simulink model "simulation_environment_thesis"  (R2024b)
settings: Solver=VariableStepAuto  StartTime=0.0  StopTime=3200  RelTol=1e-3
values from neighbouring files (symbols this model uses):
  A = [1, dt; 0, 1]    [estimate_and_control_fully_actuated_with_current.m]
  A1 = [1, 0, 0, 0, dt, 0 ... (first row only)    [estimate_and_control_fully_actuated_with_current.m]
  alpha = 10    [estimate_and_control_fully_actuated_with_current.m]
  alpha_xy_1 = 1    [estimate_and_control_fully_actuated_with_current.m]
  alpha_z_1 = 5    [estimate_and_control_fully_actuated_with_current.m]
  Az1 = [1, dt ... (first row only)    [estimate_and_control_fully_actuated_with_current.m]
  B = [dt; 0]    [estimate_and_control_fully_actuated_with_current.m]
  B1 = [dt, 0 ... (first row only)    [estimate_and_control_fully_actuated_with_current.m]
  Bz1 = [dt ... (first row only)    [estimate_and_control_fully_actuated_with_current.m]
  C1 = [1, 0, 0, 0, 0, 0 ... (first row only)    [estimate_and_control_fully_actuated_with_current.m]
  circumference_x = min_dist_from_ds*cos(theta)    [estimate_and_control_fully_actuated_with_current.m]
  circumference_y = min_dist_from_ds*sin(theta)    [estimate_and_control_fully_actuated_with_current.m]
  const_current = [-r; -s; 0; 0]    [estimate_and_control_fully_actuated_with_current.m]
  csi_psi_1 = 0.0003    [estimate_and_control_fully_actuated_with_current.m]
  csi_r_1 = 0.0003    [estimate_and_control_fully_actuated_with_current.m]
  csi_vcx_1 = 0.0003    [estimate_and_control_fully_actuated_with_current.m]
  csi_vcy_1 = 0.0003    [estimate_and_control_fully_actuated_with_current.m]
  csi_vcz_1 = 0.005    [estimate_and_control_fully_actuated_with_current.m]
  csi_x_1 = 0.003    [estimate_and_control_fully_actuated_with_current.m]
  csi_y_1 = 0.003    [estimate_and_control_fully_actuated_with_current.m]
  csi_z_1 = 0.005    [estimate_and_control_fully_actuated_with_current.m]
  Cz1 = [1, 0]    [estimate_and_control_fully_actuated_with_current.m]
  d = 1    [estimate_and_control_fully_actuated_with_current.m]
  delta_FOV = pi/7    (= 0.448798950513)    [estimate_and_control_fully_actuated_with_current.m]
  desired_speed = speed_0    [estimate_and_control_fully_actuated_with_current.m]
  dt = 1/Tsim    (= 0.005)    [estimate_and_control_fully_actuated_with_current.m]
  eta1 = deg2rad(0.5)    [estimate_and_control_fully_actuated_with_current.m]
  eta2 = deg2rad(0.5)    [estimate_and_control_fully_actuated_with_current.m]
  eta_psi_1 = 0.003    [estimate_and_control_fully_actuated_with_current.m]
  eta_x_1 = 0.03    [estimate_and_control_fully_actuated_with_current.m]
  eta_y_1 = 0.03    [estimate_and_control_fully_actuated_with_current.m]
  eta_z_1 = 0.005    [estimate_and_control_fully_actuated_with_current.m]
  G = dt*eye(2)    [estimate_and_control_fully_actuated_with_current.m]
  G1 = dt*eye(6)    [estimate_and_control_fully_actuated_with_current.m]
  Gz1 = dt*eye(2)    [estimate_and_control_fully_actuated_with_current.m]
  left_reference = L_x*[cos(NED_psi_ds); sin(NED_psi_ds); 0] + L_y_l*[-sin(NED_psi_ds); cos(NED_psi_ds); 0]    [estimate_and_control_fully_actuated_with_current.m]
  max_dist_from_ship = 60    [estimate_and_control_fully_actuated_with_current.m]
  min_dist_from_ds = 45    [estimate_and_control_fully_actuated_with_current.m]
  mu = -5    [estimate_and_control_fully_actuated_with_current.m]
  NED_psi_ds = -pi/2    (= -1.57079632679)    [estimate_and_control_fully_actuated_with_current.m]
  p_b1 = [-90; 90; 5]    [estimate_and_control_fully_actuated_with_current.m]
  position_t_0 = [0; 0; 0; 0]    [estimate_and_control_fully_actuated_with_current.m]
  psi_P = linspace(0,2*pi,360)'    [estimate_and_control_fully_actuated_with_current.m]
  Q = [csi1_kf^2, 0 ... (first row only)    [estimate_and_control_fully_actuated_with_current.m]
  Q1 = [csi_x_1_kf^2, 0, 0, 0, 0, 0 ... (first row only)    [estimate_and_control_fully_actuated_with_current.m]
  Qz1 = [csi_z_1_kf^2, 0 ... (first row only)    [estimate_and_control_fully_actuated_with_current.m]
  R = [eta1_kf^2, 0 ... (first row only)    [estimate_and_control_fully_actuated_with_current.m]
  R1 = [eta_x_1_kf^2, 0, 0 ... (first row only)    [estimate_and_control_fully_actuated_with_current.m]
  right_reference = L_x*[cos(NED_psi_ds); sin(NED_psi_ds); 0] + L_y_r*[sin(NED_psi_ds); -cos(NED_psi_ds); 0]    [estimate_and_control_fully_actuated_with_current.m]
  Rz1 = eta_z_1_kf^2    (= 1e-06)    [estimate_and_control_fully_actuated_with_current.m]
  speed_0 = [0.1, 0.1, 0, 0]'    [estimate_and_control_fully_actuated_with_current.m]
  tau_heave_sat = 30    [estimate_and_control_fully_actuated_with_current.m]
  tau_surge_sat = 10    [estimate_and_control_fully_actuated_with_current.m]
  tau_sway_sat = 30    [estimate_and_control_fully_actuated_with_current.m]
  tau_yaw_sat = 0.1    [estimate_and_control_fully_actuated_with_current.m]
  var_vm_x = 0.001    [estimate_and_control_fully_actuated_with_current.m]
  var_vm_y = 0.001    [estimate_and_control_fully_actuated_with_current.m]
  var_vm_z = 0.001    [estimate_and_control_fully_actuated_with_current.m]
undefined symbols (not defined in the file or in its folder): A2, alpha2, B2, csi1_2, csi2_2, csi3_2, csi4_2, csi5_2, csi6_2, csi7_2, csi8_2, eta1_2, eta2_2, eta3_2, eta4_2, eta5_2, eta6_2, G2, Q2, R2
libraries: simulink x60, simulink_extras x5
(part 14 of 16)

== matlab function simulation_environment_thesis/PHASE 3&4.22/PHASE  4/MATLAB Function4 ==
  data: yaw_curr input; enable output; yaw_dock input; i input; i output; yaw_dock output; enable input
function [enable, i, yaw_dock] = check_yaw_dock(yaw_curr, yaw_dock, i, enable)

    % enable = 0;
    % if abs(yaw_curr-yaw_prev)<= threshold
    %     enable = 1;
    % end


    N=100;
    N_start = 10000;
    % yaw_measured = zeros(N,1);
    if i>=N_start && i<N_start+N
        yaw_dock = yaw_curr;
        enable = 1;
    end
    % if i==N+N_start-1
    %     yaw_dock=mean(yaw_measured);
    %     enable = 1;
    % end
    i=i+1;

== matlab function simulation_environment_thesis/PHASE 3&4.22/PHASE  4/MATLAB Function5 ==
  data: angle input; angle output
function angle = normalize(angle)

angle = atan2(sin(angle), cos(angle));

== matlab function simulation_environment_thesis/PHASE 3&4.21/PHASE  3/dynamic + kinematic models1/MATLAB Function ==
  data: angle input; angle output
function angle = normalize(angle)

angle = atan2(sin(angle), cos(angle));

== matlab function simulation_environment_thesis/PHASE 3&4.21/PHASE  3/dynamic + kinematic models1/MATLAB Function7 ==
  data: speed_dot output; speed input; tau input
function speed_dot = dynamic_model(speed, tau) % speed_dot = speed_0_dot + delta_speed_dot = delta_speed_dot

    % speed and speed_dot are in the body frame
    speed_dot = zeros(4,1);
    
    W_plus_B = -0.8339;
    % m = 30;
    % I_z = 4;
    % X_du = -25;
    % Y_dv = -2;
    % Z_dw = - 20;
    % N_dr = -9;
    % X_u = -0.2;
    % Y_v = -55;
    % Z_w = 33; 
    % N_r = -4.14;
    % X_u_u = -20;
    % Y_v_v = -150;
    % Z_w_w = 90; 
    % N_r_r = -6.5;
    m = 30;
    I_z = 5;
    X_du = -25;
    Y_dv = -2;
    Z_dw = - 20;
    N_dr = -10;
    X_u = -0.2;
    Y_v = -55;
    Z_w = 35; 
    N_r = -5;
    X_u_u = -20;
    Y_v_v = -150;
    Z_w_w = 100; 
    N_r_r = -7;
    
    m_u = m - X_du;
    m_v = m - Y_dv;
    m_w = m - Z_dw;
    m_r = I_z - N_dr;
    m_u_v = m_u - m_v;
    
    % SIMPLIFIED MODEL
    
    % surge speed_dot
    speed_dot(1) = (X_u*speed(1) + X_u_u*speed(1)*abs(speed(1) + m_v*speed(2)*speed(4)) + tau(1))/m_u;
    % sway speed_dot
    speed_dot(2) = (Y_v*speed(2) + Y_v_v*speed(2)*abs(speed(2) - m_u*speed(1)*speed(4)) + tau(2))/m_v;
    % heave speed_dot
    speed_dot(3) = (-Z_w*speed(3) - Z_w_w*speed(3)*abs(speed(3)) + tau(3) + W_plus_B)/m_w;
    % yaw speed_dot
    speed_dot(4) = (N_r*speed(4) + N_r_r*speed(4)*abs(speed(4) + m_u_v*speed(1)*speed(2)) + tau(4))/m_r;

== matlab function simulation_environment_thesis/PHASE 3&4.21/PHASE  3/dynamic + kinematic models1/MATLAB Function9 ==
  data: yaw input; eta_dot output; speed input
function eta_dot = Q(yaw, speed) 
% yaw = orientation in the inertial frame, speed = robot's speed in body frame
% eta_dot = robot's speed in inertial frame

eta_dot = zeros(4,1);
R = [cos(yaw), -sin(yaw), 0;
    sin(yaw), cos(yaw), 0;
    0, 0, 1]; % z_inertial == z_body --> stesso frame, quindi la w corrisponde alla z_dot
eta_dot(1:3) = R*speed(1:3); 
eta_dot(4) = speed(4); % la yaw_dot == r di nuovo perché non ci sono né pitch né roll (stesso discorso della velocità lungo z)

== matlab function simulation_environment_thesis/PHASE 3&4.22/PHASE  4/CONTROLLER4/ANTIWINDUP SURGE/MATLAB Function21 ==
  data: in input; y output; K input
function y = fcn(in, K)
y = in*K;

== matlab function simulation_environment_thesis/PHASE 1.21/z, v_c_z estimate from DEPTH measurement/MATLAB Function8 ==
  data: K input; u input; y output
function y = fcn(K,u)

y = K*u;

== matlab function simulation_environment_thesis/PHASE 3&4.22/PHASE  4/CONTROLLER4/ANTIWINDUP SURGE/MATLAB Function22 ==
  data: in input; y output; K input
function y = fcn(in, K)
y = in*K;

== matlab function simulation_environment_thesis/PHASE 3&4.22/PHASE  4/CONTROLLER4/ANTIWINDUP SURGE/MATLAB Function23 ==
  data: e_s_u input; K_t_u input; y output
function y = AWU_surge(e_s_u, K_t_u)
y = K_t_u * e_s_u;

== matlab function simulation_environment_thesis/PHASE 3&4.22/PHASE  4/CONTROLLER4/ANTIWINDUP SWAY/MATLAB Function21 ==
  data: in input; y output; K input
function y = fcn(in, K)
y = in*K;

== matlab function simulation_environment_thesis/PHASE 3&4.22/PHASE  4/CONTROLLER4/ANTIWINDUP SWAY/MATLAB Function22 ==
  data: in input; y output; K input
function y = fcn(in, K)
y = in*K;

== matlab function simulation_environment_thesis/PHASE 3&4.22/PHASE  4/CONTROLLER4/ANTIWINDUP SWAY/MATLAB Function23 ==
  data: e_s_v input; K_t_v input; y output
function y = AWU_sway(e_s_v, K_t_v)
y = K_t_v * e_s_v;

== matlab function simulation_environment_thesis/PHASE 3&4.22/PHASE  4/CONTROLLER4/ANTIWINDUP_HEAVE/MATLAB Function11 ==
  data: e_s_w input; K_t_w input; y output
function y = AWU_heave(e_s_w, K_t_w)
y = K_t_w * e_s_w;

== matlab function simulation_environment_thesis/PHASE 3&4.22/PHASE  4/CONTROLLER4/ANTIWINDUP_HEAVE/MATLAB Function14 ==
  data: in input; y output; K input
function y = fcn(in, K)
y = in*K;

== matlab function simulation_environment_thesis/PHASE 3&4.22/PHASE  4/CONTROLLER4/ANTIWINDUP_HEAVE/MATLAB Function15 ==
  data: in input; y output; K input
function y = fcn(in, K)
y = in*K;

== matlab function simulation_environment_thesis/PHASE 3&4.22/PHASE  4/CONTROLLER4/ANTIWINDUP_HEAVE/MATLAB Function16 ==
  data: in input; y output; K input
function y = fcn(in, K)
y = in*K;

== matlab function simulation_environment_thesis/PHASE 3&4.22/PHASE  4/CONTROLLER4/ANTIWINDUP_YAW/MATLAB Function11 ==
  data: e_s_psi input; K_t_psi input; y output
function y = AWU_yaw(e_s_psi, K_t_psi)
y = K_t_psi * e_s_psi;

== matlab function simulation_environment_thesis/PHASE 1.21/z, v_c_z estimate from DEPTH measurement/MATLAB Function9 ==
  data: K_k output; P_k_k input; C input; P_k_k output; R input; A input; Q input; G input
function [K_k, P_k_k] = funct(P_k_k, C, R, A, Q, G)

P_knext_k = A*P_k_k*A' + G*Q*G';
K_k = P_knext_k*C'/(C*P_knext_k*C'+R);
P_k_k = (eye(2)-K_k*C)*P_knext_k;

== matlab function simulation_environment_thesis/PHASE 3&4.22/PHASE  4/CONTROLLER4/ANTIWINDUP_YAW/MATLAB Function14 ==
  data: in input; y output; K input
function y = fcn(in, K)
y = in*K;

== matlab function simulation_environment_thesis/PHASE 3&4.22/PHASE  4/CONTROLLER4/ANTIWINDUP_YAW/MATLAB Function15 ==
  data: in input; y output; K input
function y = fcn(in, K)
y = in*K;

== matlab function simulation_environment_thesis/PHASE 3&4.22/PHASE  4/CONTROLLER4/ANTIWINDUP_YAW/MATLAB Function16 ==
  data: in input; y output; K input
function y = fcn(in, K)
y = in*K;

== matlab function simulation_environment_thesis/PHASE 3&4.22/PHASE  4/CONTROLLER4/MATLAB Function3 ==
  data: K_i_u output; speed_0 input; K_p_u output; K_t_u output; K_i_v output; K_p_v output; K_t_v output; K_i_w output; K_p_w output; K_d_w output; K_t_w output; K_p_psi output; K_i_psi output; K_d_psi output; K_t_psi output
function [K_i_u, K_p_u, K_t_u, K_i_v, K_p_v, K_t_v, K_i_w, K_p_w, K_d_w, K_t_w, K_p_psi, K_i_psi, K_d_psi, K_t_psi] = computeGains(speed_0)
csi = 0.6;
omega_n = 0.2;
p = 10; % 10 o -10?

m = 30;
I_z = 4.14;
X_du = -25;
Y_dv = -2.325;
Z_dw = -19.4311;
N_dr = -8.690;
X_u = -0.2;
Y_v = -55.117;
Z_w = 33.6804; % CONTROLLA SEGNO con - viene positivo K_p, con + viene neg. dovrebbe essere positivo perché le eq sono al contrario (????)
X_u_u = -19.5;
Y_v_v = -147.9;
Z_w_w = 89.4105; % CONTROLLA SEGNO
N_r = -4.14;
N_r_r = -6.23;
m_u = m - X_du;
m_v = m - Y_dv;
m_w = m - Z_dw;
m_r = I_z - N_dr;

K_i_u = omega_n^2*m_u; %2
K_p_u = 2*csi*omega_n*m_u + X_u + X_u_u*2*abs(speed_0(1)); %9
t_i_u = K_p_u/K_i_u;
K_t_u = 1/t_i_u;


K_i_v = m_v*omega_n^2;
K_p_v = 2*csi*omega_n*m_v + Y_v + 2*Y_v_v*abs(speed_0(2));
t_i_v = K_p_v/K_i_v;
K_t_v = 1/t_i_v;

% PI SULLA VELOCITà SBAGLIATO
% K_i_w = m_w*omega_n^2; %2
% K_p_w = 2*csi*omega_n*m_w - Z_w - Z_w_w*2*abs(speed_0(3)); %negativo, -22
% t_i_w = K_p_w/K_i_w;
% K_t_w = 1/t_i_w;

% PID SULLA POSIZIONE
K_i_w = m_w*p*omega_n^2; % 19.7724
K_p_w = m_w*(omega_n^2 + 2*csi*omega_n*p); % 120.6119
K_d_w = m_w*(p + 2*csi*omega_n) - Z_w - Z_w_w*2*abs(speed_0(3)); % 472.4941
t_i_w = K_p_w/K_i_w;
t_d_w = K_d_w/K_p_w;
K_t_w = 1/sqrt(t_i_w*t_d_w);

K_p_psi = m_r*(omega_n^2 + 2*csi*omega_n*p); %31
K_i_psi = m_r*p*omega_n^2; %5
K_d_psi = m_r*(2*csi*omega_n + p) + N_r + N_r_r*2*abs(speed_0(4));
t_i_psi = K_p_psi/K_i_psi;
t_d_psi = K_d_psi/K_p_psi;
K_t_psi = 1/sqrt(t_i_psi*t_d_psi);

== matlab function simulation_environment_thesis/PHASE 3&4.22/PHASE  4/MATLAB Function ==
  data: ship_p_auv input; ds_p_auv output; ship_p_ds input
function ds_p_auv = coord_transf(ship_p_auv, ship_p_ds)


ds_p_auv = ship_p_auv - ship_p_ds;

== matlab function simulation_environment_thesis/PHASE 3&4.22/PHASE  4/MATLAB Function1 ==
  data: ship_p_auv output; ds_p_auv input; ship_p_ds input
function ship_p_auv = transform(ds_p_auv, ship_p_ds)
 

ship_p_auv = ship_p_ds + ds_p_auv;

== matlab function simulation_environment_thesis/PHASE 3&4.22/PHASE  4/MATLAB Function10 ==
  data: angle input; angle output
function angle = normalize(angle)

angle = atan2(sin(angle), cos(angle));

== matlab function simulation_environment_thesis/PHASE 3&4  /PHASE  4/dynamic + kinematic models1/MATLAB Function ==
  data: angle input; angle output
function angle = normalize(angle)

angle = atan2(sin(angle), cos(angle));

== matlab function simulation_environment_thesis/PHASE 3&4.22/PHASE  4/MATLAB Function18 ==
  data: yaw_err input; yaw_dock output; yaw_curr input
function yaw_dock = compute(yaw_err, yaw_curr)
    
    yaw_dock = yaw_curr - yaw_err;
    %yaw_dock = atan2(sin(yaw_dock), cos(yaw_dock));

== matlab function simulation_environment_thesis/PHASE 3&4  /PHASE  4/dynamic + kinematic models1/MATLAB Function7 ==
  data: speed_dot output; speed input; tau input
function speed_dot = dynamic_model(speed, tau) % speed_dot = speed_0_dot + delta_speed_dot = delta_speed_dot

    % speed and speed_dot are in the body frame
    speed_dot = zeros(4,1);
    
    W_plus_B = -0.8339;
    % m = 30;
    % I_z = 4;
    % X_du = -25;
    % Y_dv = -2;
    % Z_dw = - 20;
    % N_dr = -9;
    % X_u = -0.2;
    % Y_v = -55;
    % Z_w = 33; 
    % N_r = -4.14;
    % X_u_u = -20;
    % Y_v_v = -150;
    % Z_w_w = 90; 
    % N_r_r = -6.5;
    m = 30;
    I_z = 5;
    X_du = -25;
    Y_dv = -2;
    Z_dw = - 20;
    N_dr = -10;
    X_u = -0.2;
    Y_v = -55;
    Z_w = 35; 
    N_r = -5;
    X_u_u = -20;
    Y_v_v = -150;
    Z_w_w = 100; 
    N_r_r = -7;
    
    m_u = m - X_du;
    m_v = m - Y_dv;
    m_w = m - Z_dw;
    m_r = I_z - N_dr;
    m_u_v = m_u - m_v;
    
    % SIMPLIFIED MODEL
    
    % surge speed_dot
    speed_dot(1) = (X_u*speed(1) + X_u_u*speed(1)*abs(speed(1) + m_v*speed(2)*speed(4)) + tau(1))/m_u;
    % sway speed_dot
    speed_dot(2) = (Y_v*speed(2) + Y_v_v*speed(2)*abs(speed(2) - m_u*speed(1)*speed(4)) + tau(2))/m_v;
    % heave speed_dot
    speed_dot(3) = (-Z_w*speed(3) - Z_w_w*speed(3)*abs(speed(3)) + tau(3) + W_plus_B)/m_w;
    % yaw speed_dot
    speed_dot(4) = (N_r*speed(4) + N_r_r*speed(4)*abs(speed(4) + m_u_v*speed(1)*speed(2)) + tau(4))/m_r;

== matlab function simulation_environment_thesis/PHASE 3&4.22/PHASE  4/MATLAB Function6 ==
  data: angle input; angle output
function angle = normalize(angle)

angle = atan2(sin(angle), cos(angle));

== matlab function simulation_environment_thesis/PHASE 3&4.22/PHASE  4/MATLAB Function7 ==
  data: v_line input; v_blend output; v_circ input; w input
function v_blend = blend_vel(v_line, v_circ, w)

% K_perp = 0.5;
% t = [cos(NED_psi_dock); sin(NED_psi_dock)];
% n = [-t(2); t(1)];


v_blend = (1 - w) * v_circ + v_line;

== matlab function simulation_environment_thesis/PHASE 3&4.22/PHASE  4/MATLAB Function9 ==
  data: ds_p_auv input; docked output; ds_p_ds input; docked input; range output
function [docked, range] = check_distance(ds_p_auv, ds_p_ds, docked)
    
    threshold = 0.3; % 10 cm of threshold, but we can vary
    range = norm(ds_p_ds - ds_p_auv);
    if docked == 1
        return;
    end
    
    if range <= threshold
        docked = 1;
    end

== matlab function simulation_environment_thesis/PHASE 3&4  /PHASE  4/dynamic + kinematic models1/MATLAB Function9 ==
  data: yaw input; eta_dot output; speed input
function eta_dot = Q(yaw, speed) 
% yaw = orientation in the inertial frame, speed = robot's speed in body frame
% eta_dot = robot's speed in inertial frame

eta_dot = zeros(4,1);
R = [cos(yaw), -sin(yaw), 0;
    sin(yaw), cos(yaw), 0;
    0, 0, 1]; % z_inertial == z_body --> stesso frame, quindi la w corrisponde alla z_dot
eta_dot(1:3) = R*speed(1:3); 
eta_dot(4) = speed(4); % la yaw_dot == r di nuovo perché non ci sono né pitch né roll (stesso discorso della velocità lungo z)

== matlab function simulation_environment_thesis/PHASE 3&4.22/PHASE  4/Subsystem/MATLAB Function1 ==
  data: radius input; stop_flag output; NED_psi_dock input; v_c input; t_trans input; x_y input; stop_flag input; ds_Pstart output; ds_Pstart_prev output; dist output
function [stop_flag, ds_Pstart, ds_Pstart_prev, dist] = check_distance(radius, NED_psi_dock, v_c, t_trans, x_y, stop_flag)
    
    threshold = 5;
    NED_psi_ds = NED_psi_dock - pi;
    ds_Pstart_prev = radius*[cos(NED_psi_ds); sin(NED_psi_ds)];
    ds_drift = v_c*t_trans;
    ds_Pstart = ds_Pstart_prev - ds_drift;
    dist = ds_Pstart - x_y;
    dist = abs(dist);
    if stop_flag == 1
        return;
    end
    
    if dist <= threshold*ones(2,1)
        stop_flag = 1;
    end

== matlab function simulation_environment_thesis/PHASE 1.22/CONTROLLER1/ANTIWINDUP SURGE/MATLAB Function21 ==
  data: in input; y output; K input
function y = fcn(in, K)
y = in*K;

== matlab function simulation_environment_thesis/PHASE 3&4.22/PHASE  4/Subsystem/MATLAB Function2 ==
  data: right_trans input; stop_flag output; left_trans input; ds_p_auv input; stop_flag input; P_R_range output; P_L_range output
function [stop_flag, P_R_range, P_L_range] = check_distance(right_trans, left_trans, ds_p_auv, stop_flag)
    
    
    threshold = 0.01; % vediamo se basta
    P_R_range = norm(right_trans(1:2) - ds_p_auv);
    P_L_range = norm(left_trans(1:2) - ds_p_auv);
    if stop_flag == 1
        return;
    end
    if abs(P_R_range - P_L_range) <= threshold
        stop_flag = 1;
    end

== matlab function simulation_environment_thesis/PHASE 3&4.22/PHASE  4/Subsystem/MATLAB Function3 ==
  data: v_tot_des input; v_c input; psi_dock input; speed0 input; speed_desired output; beta input
function speed_desired = circ_guidance(v_tot_des, v_c, psi_dock, speed0, beta)
    v_c_x = v_c(1);
    v_c_y = v_c(2);
    
    
    
    u = cos(psi_dock)*(v_tot_des*cos(beta) - v_c_x) + sin(psi_dock)*(v_tot_des*sin(beta) - v_c_y);
    v = -sin(psi_dock)*(v_tot_des*cos(beta) - v_c_x) + cos(psi_dock)*(v_tot_des*sin(beta) - v_c_y);
    speed_desired = [u; v; speed0(3:4)];

== matlab function simulation_environment_thesis/PHASE 3&4.22/PHASE  4/Subsystem/MATLAB Function4 ==
  data: NED_psi_dock input; t output
function t = tang(NED_psi_dock)

    t = [cos(NED_psi_dock); sin(NED_psi_dock)];

== matlab function simulation_environment_thesis/PHASE 1.22/dynamic + kinematic models1/MATLAB Function ==
  data: angle input; angle output
function angle = normalize(angle)

angle = atan2(sin(angle), cos(angle));

== matlab function simulation_environment_thesis/PHASE 1.22/dynamic + kinematic models1/MATLAB Function7 ==
  data: speed_dot output; speed input; tau input
function speed_dot = dynamic_model(speed, tau) % speed_dot = speed_0_dot + delta_speed_dot = delta_speed_dot

    % speed and speed_dot are in the body frame
    speed_dot = zeros(4,1);
    
    W_plus_B = -0.8339;
    % m = 30;
    % I_z = 4;
    % X_du = -25;
    % Y_dv = -2;
    % Z_dw = - 20;
    % N_dr = -9;
    % X_u = -0.2;
    % Y_v = -55;
    % Z_w = 33; 
    % N_r = -4.14;
    % X_u_u = -20;
    % Y_v_v = -150;
    % Z_w_w = 90; 
    % N_r_r = -6.5;
    m = 30;
    I_z = 5;
    X_du = -25;
    Y_dv = -2;
    Z_dw = - 20;
    N_dr = -10;
    X_u = -0.2;
    Y_v = -55;
    Z_w = 35; 
    N_r = -5;
    X_u_u = -20;
    Y_v_v = -150;
    Z_w_w = 100; 
    N_r_r = -7;
    
    m_u = m - X_du;
    m_v = m - Y_dv;
    m_w = m - Z_dw;
    m_r = I_z - N_dr;
    m_u_v = m_u - m_v;
    
    % SIMPLIFIED MODEL
    
    % surge speed_dot
    speed_dot(1) = (X_u*speed(1) + X_u_u*speed(1)*abs(speed(1) + m_v*speed(2)*speed(4)) + tau(1))/m_u;
    % sway speed_dot
    speed_dot(2) = (Y_v*speed(2) + Y_v_v*speed(2)*abs(speed(2) - m_u*speed(1)*speed(4)) + tau(2))/m_v;
    % heave speed_dot
    speed_dot(3) = (-Z_w*speed(3) - Z_w_w*speed(3)*abs(speed(3)) + tau(3) + W_plus_B)/m_w;
    % yaw speed_dot
    speed_dot(4) = (N_r*speed(4) + N_r_r*speed(4)*abs(speed(4) + m_u_v*speed(1)*speed(2)) + tau(4))/m_r;

== matlab function simulation_environment_thesis/PHASE 3&4.22/PHASE  4/Subsystem/MATLAB Function7 ==
  data: eta input; circ_x input; circ_y input; psi_P input; beta input; direction output; flag output; psi_P output; i output
function [direction, flag, psi_P, i]= closest_pnt(eta, circ_x, circ_y, psi_P, beta)
    n = size(circ_x,1);
    p_r = eta(1:2, 1); % OK
    eucl_dist = zeros(n,1);
    for j = 1:n
        eucl_dist(j) = sqrt((p_r(1)-circ_x(j))^2+(p_r(2)-circ_y(j))^2);
    end
    [min_dist, i] = min(eucl_dist);
    flag = 0; % to see if there's a 2pi gap between the previous psi and the current one

    if abs(psi_P(i)-beta)> 2*pi
        flag = 1;
        psi_P = psi_P - 2*pi; % controlla la situa con - o col +, potrebbe esserci un problema se non devo sottrarre ma sommare 2pi
    end
    direction = psi_P(i) - pi/2;
    direction = atan2(sin(direction), cos(direction));
    end

== matlab function simulation_environment_thesis/PHASE 3&4.22/PHASE  4/MATLAB Function8 ==
  data: u input; y output
function y = fcn(u)

y = u;
if abs(u-2*pi)<=0.01
    y = u-2*pi;
elseif abs(u+2*pi)<=0.01
    y = u+2*pi;
end

== matlab function simulation_environment_thesis/PHASE 1.24/dynamic + kinematic models1/MATLAB Function ==
  data: angle input; angle output
function angle = normalize(angle)

angle = atan2(sin(angle), cos(angle));

== matlab function simulation_environment_thesis/PHASE 1.22/CONTROLLER1/ANTIWINDUP SURGE/MATLAB Function22 ==
  data: in input; y output; K input
function y = fcn(in, K)
y = in*K;

== matlab function simulation_environment_thesis/PHASE 1.24/dynamic + kinematic models1/MATLAB Function7 ==
  data: speed_dot output; speed input; tau input
function speed_dot = dynamic_model(speed, tau) % speed_dot = speed_0_dot + delta_speed_dot = delta_speed_dot

    % speed and speed_dot are in the body frame
    speed_dot = zeros(4,1);
    
    W_plus_B = -0.8339;
    % m = 30;
    % I_z = 4;
    % X_du = -25;
    % Y_dv = -2;
    % Z_dw = - 20;
    % N_dr = -9;
    % X_u = -0.2;
    % Y_v = -55;
    % Z_w = 33; 
    % N_r = -4.14;
    % X_u_u = -20;
    % Y_v_v = -150;
    % Z_w_w = 90; 
    % N_r_r = -6.5;
    m = 30;
    I_z = 5;
    X_du = -25;
    Y_dv = -2;
    Z_dw = - 20;
    N_dr = -10;
    X_u = -0.2;
    Y_v = -55;
    Z_w = 35; 
    N_r = -5;
    X_u_u = -20;
    Y_v_v = -150;
    Z_w_w = 100; 
    N_r_r = -7;
    
    m_u = m - X_du;
    m_v = m - Y_dv;
    m_w = m - Z_dw;
    m_r = I_z - N_dr;
    m_u_v = m_u - m_v;
    
    % SIMPLIFIED MODEL
    
    % surge speed_dot
    speed_dot(1) = (X_u*speed(1) + X_u_u*speed(1)*abs(speed(1) + m_v*speed(2)*speed(4)) + tau(1))/m_u;
    % sway speed_dot
    speed_dot(2) = (Y_v*speed(2) + Y_v_v*speed(2)*abs(speed(2) - m_u*speed(1)*speed(4)) + tau(2))/m_v;
    % heave speed_dot
    speed_dot(3) = (-Z_w*speed(3) - Z_w_w*speed(3)*abs(speed(3)) + tau(3) + W_plus_B)/m_w;
    % yaw speed_dot
    speed_dot(4) = (N_r*speed(4) + N_r_r*speed(4)*abs(speed(4) + m_u_v*speed(1)*speed(2)) + tau(4))/m_r;

== matlab function simulation_environment_thesis/PHASE 3&4.22/PHASE  4/p, v_c, psi, r (3D) estimate, from RANGE, v_c, PSI measurement/MATLAB Function ==
  data: p input; y output
function y = range(p)
    y = sqrt(p(1)^2 + p(2)^2 + p(3)^2);

== matlab function simulation_environment_thesis/PHASE 3&4.22/PHASE  4/p, v_c, psi, r (3D) estimate, from RANGE, v_c, PSI measurement/MATLAB Function10 ==
  data: K_knext output; P_k_k input; P_knext_knext output; R input; A input; Q input; G input; x_k_kprev input
function [K_knext, P_knext_knext] = funct(P_k_k, R, A, Q, G, x_k_kprev)

P_knext_k = A*P_k_k*A' + G*Q*G';
H = zeros(6,8);
H(1,1) = x_k_kprev(1)/sqrt(x_k_kprev(1)^2+x_k_kprev(2)^2+x_k_kprev(3)^2);
H(1,2) = x_k_kprev(2)/sqrt(x_k_kprev(1)^2+x_k_kprev(2)^2+x_k_kprev(3)^2);
H(1,3) = x_k_kprev(3)/sqrt(x_k_kprev(1)^2+x_k_kprev(2)^2+x_k_kprev(3)^2);
H(2,4) = 1;
H(3,5) = 1;
H(4,6) = 1;
H(5,7) = 1;
H(6,3) = 1;
K_knext = P_knext_k*H'/(H*P_knext_k*H'+R);
P_knext_knext = (eye(8)-K_knext*H)*P_knext_k;

== matlab function simulation_environment_thesis/PHASE 3&4.22/PHASE  4/p, v_c, psi, r (3D) estimate, from RANGE, v_c, PSI measurement/MATLAB Function6 ==
  data: x_k_kprev input; y output
function y = pred(x_k_kprev)

y = zeros(6,1);
y(1) =sqrt(x_k_kprev(1)^2 + x_k_kprev(2)^2 + x_k_kprev(3)^2);
y(2:5) = x_k_kprev(4:7);
y(6) = x_k_kprev(3);

== matlab function simulation_environment_thesis/PHASE 3&4.22/PHASE  4/p, v_c, psi, r (3D) estimate, from RANGE, v_c, PSI measurement/MATLAB Function7 ==
  data: A input; u input; x_k_k output
function x_k_k = fcn(A,u)

x_k_k = A*u;

== matlab function simulation_environment_thesis/PHASE 3&4.22/PHASE  4/p, v_c, psi, r (3D) estimate, from RANGE, v_c, PSI measurement/MATLAB Function8 ==
  data: B input; u input; y output
function y = fcn(B,u)

y = B*u;

== matlab function simulation_environment_thesis/PHASE 3&4.22/PHASE  4/p, v_c, psi, r (3D) estimate, from RANGE, v_c, PSI measurement/MATLAB Function9 ==
  data: K input; y_err input; y output
function y = err(K,y_err)

y = K*y_err;

== matlab function simulation_environment_thesis/PHASE 3&4.22/PHASE  4/positon and current estimate, XY PLANE from X, Y, psi measurement/MATLAB Function10 ==
  data: K_knext output; P_k_k input; P_knext_knext output; R input; A input; Q input; G input; C input
function [K_knext, P_knext_knext] = funct(P_k_k, R, A, Q, G, C)

P_knext_k = A*P_k_k*A' + G*Q*G';
K_knext = P_knext_k*C'/(C*P_knext_k*C'+R);
P_knext_knext = (eye(6)-K_knext*C)*P_knext_k;

== matlab function simulation_environment_thesis/PHASE 3&4.22/PHASE  4/positon and current estimate, XY PLANE from X, Y, psi measurement/MATLAB Function6 ==
  data: x_k_kprev input; pred output; C input
function pred = fcn(x_k_kprev, C)
pred = C*x_k_kprev;

== matlab function simulation_environment_thesis/PHASE 1.22/CONTROLLER1/ANTIWINDUP SURGE/MATLAB Function23 ==
  data: e_s_u input; K_t_u input; y output
function y = AWU_surge(e_s_u, K_t_u)
y = K_t_u * e_s_u;

== matlab function simulation_environment_thesis/PHASE 3&4.22/PHASE  4/positon and current estimate, XY PLANE from X, Y, psi measurement/MATLAB Function7 ==
  data: A input; u input; x_k_k output
function x_k_k = fcn(A,u)

x_k_k = A*u;

== matlab function simulation_environment_thesis/PHASE 3&4.22/PHASE  4/positon and current estimate, XY PLANE from X, Y, psi measurement/MATLAB Function8 ==
  data: B input; u input; y output
function y = fcn(B,u)

y = B*u;

== matlab function simulation_environment_thesis/PHASE 3&4.22/PHASE  4/positon and current estimate, XY PLANE from X, Y, psi measurement/MATLAB Function9 ==
  data: K input; y_err input; y output
function y = fcn(K,y_err)

y = K*y_err;

== matlab function simulation_environment_thesis/PHASE 3&4.22/PHASE  4/relative yaw//bias estimate from VECTOR measurement1/MATLAB Function12 ==
  data: d input; psi input; y output
function y = output_est(d, psi)
 
y = zeros(2,1);
y(1) = - d*sin(psi);
y(2) = d*cos(psi);

== matlab function simulation_environment_thesis/PHASE 3&4.22/PHASE  4/relative yaw//bias estimate from VECTOR measurement1/MATLAB Function13 ==
  data: K_k output; P_k_k input; R input; A input; Q input; G input; x_k_kprev input; P_k_k output; d input
function [K_k, P_k_k] = funct(P_k_k, R, A, Q, G, x_k_kprev, d)

P_knext_k = A*P_k_k*A' + G*Q*G';
H = zeros(2);
H(1,1) = - d*cos(x_k_kprev(1));
H(2,1) = - d*sin(x_k_kprev(1));

K_k = P_knext_k*H'/(H*P_knext_k*H'+R);
P_k_k = (eye(2)-K_k*H)*P_knext_k;

== matlab function simulation_environment_thesis/PHASE 3&4.22/PHASE  4/relative yaw//bias estimate from VECTOR measurement1/MATLAB Function14 ==
  data: A input; u input; y output
function y = fcn(A,u)

y = A*u;

== matlab function simulation_environment_thesis/PHASE 3&4.22/PHASE  4/relative yaw//bias estimate from VECTOR measurement1/MATLAB Function15 ==
  data: K input; u input; y output
function y = err(K,u)

y = K*u;

== matlab function simulation_environment_thesis/PHASE 3&4.22/PHASE  4/relative yaw//bias estimate from VECTOR measurement1/MATLAB Function16 ==
  data: B input; u input; y output
function y = fcn(B,u)

y = B*u;

== matlab function simulation_environment_thesis/PHASE 3&4.22/PHASE  4/relative yaw//bias estimate from VECTOR measurement1/MATLAB Function3 ==
  data: psi input; y output; d input
function y = real_obs(psi, d)

y = zeros(2,1);
y(1) = - d*sin(psi);
y(2) = d*cos(psi);

== matlab function simulation_environment_thesis/PHASE 1.22/CONTROLLER1/ANTIWINDUP SWAY/MATLAB Function21 ==
  data: in input; y output; K input
function y = fcn(in, K)
y = in*K;

== matlab function simulation_environment_thesis/PHASE 3&4.22/Subsystem3/MATLAB Function7 ==
  data: num input; out output
function out = phase(num)

    out = NaN;
    if num == 1
        out = 0;
    elseif num == 2
        out = 1;
    end

== matlab function simulation_environment_thesis/PHASE 3&4.22/end phase 3/# of pings ==
  data: alpha_ds input; num_of_pings output; delta input; ds_p_ds input; ds_p_auv input
function num_of_pings = sensor(alpha_ds, delta, ds_p_ds, ds_p_auv)

    dir = [cos(alpha_ds), sin(alpha_ds)]; % main direction of the DS opening cone
    distance = ds_p_auv(1:2) - ds_p_ds; % vector connecting the center of the DS to the AUV
    dot_prod = dot(distance,dir) / norm(distance);

    in_cone = (dot_prod >= cos(delta)) & (norm(distance) > 0);

    if in_cone == 0 % go to PHASE 3
        num_of_pings = 1; %% come gestisco quando ne tornano 0? ne possono tornare 0?
    else % go to PHASE 4
        num_of_pings = 2;
    end

== matlab function simulation_environment_thesis/PHASE 3&4.22/logic to recognize where i am, based on the # of pings/MATLAB Function6 ==
  data: num input; out output
function out = phase(num)
    out = 0;
    if num == 1
        out = 0;
    elseif num == 2
        out = 1;
    end

== matlab function simulation_environment_thesis/PHASE 3&4.22/simulator of pings from sensor/# of pings ==
  data: alpha_ds input; num_of_pings output; delta input; ds_p_ds input; ds_p_auv input; stop_flag input
function num_of_pings = sensor(alpha_ds, delta, ds_p_ds, ds_p_auv, stop_flag)
    if stop_flag == 0 
        num_of_pings = NaN;
        return;
    end

    dir = [cos(alpha_ds), sin(alpha_ds)]; % main direction of the DS opening cone
    distance = ds_p_auv(1:2) - ds_p_ds; % vector connecting the center of the DS to the AUV
    dot_prod = dot(distance,dir) / norm(distance);

    in_cone = (dot_prod >= cos(delta)) & (norm(distance) > 0);

    if in_cone == 0 % go to PHASE 3
        num_of_pings = 1; %% come gestisco quando ne tornano 0? ne possono tornare 0?
    else % go to PHASE 4
        num_of_pings = 2;
    end

== matlab function simulation_environment_thesis/PHASE 3&4  /PHASE  3/CONTROLLER/ANTIWINDUP SURGE/MATLAB Function21 ==
  data: in input; y output; K input
function y = fcn(in, K)
y = in*K;

== matlab function simulation_environment_thesis/PHASE 3&4  /PHASE  3/CONTROLLER/ANTIWINDUP SURGE/MATLAB Function22 ==
  data: in input; y output; K input
function y = fcn(in, K)
y = in*K;

== matlab function simulation_environment_thesis/PHASE 3&4  /PHASE  3/CONTROLLER/ANTIWINDUP SURGE/MATLAB Function23 ==
  data: e_s_u input; K_t_u input; y output
function y = AWU_surge(e_s_u, K_t_u)
y = K_t_u * e_s_u;

== matlab function simulation_environment_thesis/PHASE 3&4  /PHASE  3/CONTROLLER/ANTIWINDUP SWAY/MATLAB Function21 ==
  data: in input; y output; K input
function y = fcn(in, K)
y = in*K;

== matlab function simulation_environment_thesis/PHASE 3&4  /PHASE  3/CONTROLLER/ANTIWINDUP SWAY/MATLAB Function22 ==
  data: in input; y output; K input
function y = fcn(in, K)
y = in*K;

== matlab function simulation_environment_thesis/PHASE 3&4  /PHASE  3/CONTROLLER/ANTIWINDUP SWAY/MATLAB Function23 ==
  data: e_s_v input; K_t_v input; y output
function y = AWU_sway(e_s_v, K_t_v)
y = K_t_v * e_s_v;

== matlab function simulation_environment_thesis/PHASE 1.22/CONTROLLER1/ANTIWINDUP SWAY/MATLAB Function22 ==
  data: in input; y output; K input
function y = fcn(in, K)
y = in*K;

== matlab function simulation_environment_thesis/PHASE 3&4  /PHASE  3/CONTROLLER/ANTIWINDUP_HEAVE/MATLAB Function11 ==
  data: e_s_w input; K_t_w input; y output
function y = AWU_heave(e_s_w, K_t_w)
y = K_t_w * e_s_w;

== matlab function simulation_environment_thesis/PHASE 3&4  /PHASE  3/CONTROLLER/ANTIWINDUP_HEAVE/MATLAB Function14 ==
  data: in input; y output; K input
function y = fcn(in, K)
y = in*K;

== matlab function simulation_environment_thesis/PHASE 3&4  /PHASE  3/CONTROLLER/ANTIWINDUP_HEAVE/MATLAB Function15 ==
  data: in input; y output; K input
function y = fcn(in, K)
y = in*K;

== matlab function simulation_environment_thesis/PHASE 3&4  /PHASE  3/CONTROLLER/ANTIWINDUP_HEAVE/MATLAB Function16 ==
  data: in input; y output; K input
function y = fcn(in, K)
y = in*K;

== matlab function simulation_environment_thesis/PHASE 3&4  /PHASE  3/CONTROLLER/ANTIWINDUP_YAW/MATLAB Function11 ==
  data: e_s_psi input; K_t_psi input; y output
function y = AWU_yaw(e_s_psi, K_t_psi)
y = K_t_psi * e_s_psi;

== matlab function simulation_environment_thesis/PHASE 3&4  /PHASE  3/CONTROLLER/ANTIWINDUP_YAW/MATLAB Function14 ==
  data: in input; y output; K input
function y = fcn(in, K)
y = in*K;

== matlab function simulation_environment_thesis/PHASE 3&4  /PHASE  3/CONTROLLER/ANTIWINDUP_YAW/MATLAB Function15 ==
  data: in input; y output; K input
function y = fcn(in, K)
y = in*K;

== matlab function simulation_environment_thesis/PHASE 3&4  /PHASE  3/CONTROLLER/ANTIWINDUP_YAW/MATLAB Function16 ==
  data: in input; y output; K input
function y = fcn(in, K)
y = in*K;

== matlab function simulation_environment_thesis/PHASE 3&4  /PHASE  3/CONTROLLER/MATLAB Function3 ==
  data: K_i_u output; speed_0 input; K_p_u output; K_t_u output; K_i_v output; K_p_v output; K_t_v output; K_i_w output; K_p_w output; K_d_w output; K_t_w output; K_p_psi output; K_i_psi output; K_d_psi output; K_t_psi output
function [K_i_u, K_p_u, K_t_u, K_i_v, K_p_v, K_t_v, K_i_w, K_p_w, K_d_w, K_t_w, K_p_psi, K_i_psi, K_d_psi, K_t_psi] = computeGains(speed_0)
csi = 0.6;
omega_n = 0.2;
p = 10; % 10 o -10?

m = 30;
I_z = 4.14;
X_du = -25;
Y_dv = -2.325;
Z_dw = -19.4311;
N_dr = -8.690;
X_u = -0.2;
Y_v = -55.117;
Z_w = 33.6804; % CONTROLLA SEGNO con - viene positivo K_p, con + viene neg. dovrebbe essere positivo perché le eq sono al contrario (????)
X_u_u = -19.5;
Y_v_v = -147.9;
Z_w_w = 89.4105; % CONTROLLA SEGNO
N_r = -4.14;
N_r_r = -6.23;
m_u = m - X_du;
m_v = m - Y_dv;
m_w = m - Z_dw;
m_r = I_z - N_dr;

K_i_u = omega_n^2*m_u; %2
K_p_u = 2*csi*omega_n*m_u + X_u + X_u_u*2*abs(speed_0(1)); %9
t_i_u = K_p_u/K_i_u;
K_t_u = 1/t_i_u;


K_i_v = m_v*omega_n^2;
K_p_v = 2*csi*omega_n*m_v + Y_v + 2*Y_v_v*abs(speed_0(2));
t_i_v = K_p_v/K_i_v;
K_t_v = 1/t_i_v;

% PI SULLA VELOCITà SBAGLIATO
% K_i_w = m_w*omega_n^2; %2
% K_p_w = 2*csi*omega_n*m_w - Z_w - Z_w_w*2*abs(speed_0(3)); %negativo, -22
% t_i_w = K_p_w/K_i_w;
% K_t_w = 1/t_i_w;

% PID SULLA POSIZIONE
K_i_w = m_w*p*omega_n^2; % 19.7724
K_p_w = m_w*(omega_n^2 + 2*csi*omega_n*p); % 120.6119
K_d_w = m_w*(p + 2*csi*omega_n) - Z_w - Z_w_w*2*abs(speed_0(3)); % 472.4941
t_i_w = K_p_w/K_i_w;
t_d_w = K_d_w/K_p_w;
K_t_w = 1/sqrt(t_i_w*t_d_w);

K_p_psi = m_r*(omega_n^2 + 2*csi*omega_n*p); %31
K_i_psi = m_r*p*omega_n^2; %5
K_d_psi = m_r*(2*csi*omega_n + p) + N_r + N_r_r*2*abs(speed_0(4));
t_i_psi = K_p_psi/K_i_psi;
t_d_psi = K_d_psi/K_p_psi;
K_t_psi = 1/sqrt(t_i_psi*t_d_psi);

== matlab function simulation_environment_thesis/PHASE 1.22/CONTROLLER1/ANTIWINDUP SWAY/MATLAB Function23 ==
  data: e_s_v input; K_t_v input; y output
function y = AWU_sway(e_s_v, K_t_v)
y = K_t_v * e_s_v;

== matlab function simulation_environment_thesis/PHASE 3&4  /PHASE  3/MATLAB Function ==
  data: ship_p_auv input; ds_p_auv output; ship_p_ds input
function ds_p_auv = coord_transf(ship_p_auv, ship_p_ds)


ds_p_auv = ship_p_auv - ship_p_ds;

== matlab function simulation_environment_thesis/PHASE 3&4  /PHASE  3/MATLAB Function1 ==
  data: ship_p_auv output; ds_p_auv input; ship_p_ds input
function ship_p_auv = fcn(ds_p_auv, ship_p_ds)
 

ship_p_auv = ship_p_ds + ds_p_auv;

== matlab function simulation_environment_thesis/PHASE 1.22/dynamic + kinematic models1/MATLAB Function9 ==
  data: yaw input; eta_dot output; speed input
function eta_dot = Q(yaw, speed) 
% yaw = orientation in the inertial frame, speed = robot's speed in body frame
% eta_dot = robot's speed in inertial frame

eta_dot = zeros(4,1);
R = [cos(yaw), -sin(yaw), 0;
    sin(yaw), cos(yaw), 0;
    0, 0, 1]; % z_inertial == z_body --> stesso frame, quindi la w corrisponde alla z_dot
eta_dot(1:3) = R*speed(1:3); 
eta_dot(4) = speed(4); % la yaw_dot == r di nuovo perché non ci sono né pitch né roll (stesso discorso della velocità lungo z)

== matlab function simulation_environment_thesis/PHASE 3&4  /PHASE  3/REFERENCE GENERATOR/MATLAB Function2 ==
  data: yaw_des input; yaw_des output
function yaw_des = normalize(yaw_des)

yaw_des = atan2(sin(yaw_des), cos(yaw_des));

== matlab function simulation_environment_thesis/PHASE 3&4  /PHASE  3/REFERENCE GENERATOR/MATLAB Function3 ==
  data: psi_p input; psi output; y_1 input; delta_h input
function psi = heading_ctrl_law(psi_p, y_1, delta_h)
    psi = psi_p + atan2(y_1,delta_h);
    % no -y_1 because of how I built y_1

== matlab function simulation_environment_thesis/PHASE 3&4  /PHASE  3/REFERENCE GENERATOR/MATLAB Function4 ==
  data: y_1 output; eta input; circ_x input; circ_y input; center input; radius input; psi_P input; prev_psi_P input; direction output; flag output; psi_P output; i output
function [y_1, direction, flag, psi_P, i]= closest_pnt(eta, circ_x, circ_y, center, radius, psi_P, prev_psi_P)
    n = size(circ_x,1);
    p_r = eta(1:2, 1); % OK
    eucl_dist = zeros(n,1);
    for j = 1:n
        eucl_dist(j) = sqrt((p_r(1)-circ_x(j))^2+(p_r(2)-circ_y(j))^2);
    end
    [min_dist, i] = min(eucl_dist);


    closest = [circ_x(i); circ_y(i)]; % OK
    y_1_I = p_r - closest; % OK
    y_1 = sqrt(y_1_I(1)^2 + y_1_I(2)^2); % OK
    sign = 1;
    dist = p_r - center;
    dist_mod = sqrt(dist(1)^2 + dist(2)^2);
    if dist_mod > radius
        sign = -1;
    elseif abs(dist_mod - radius) < 0.01
        sign = 0;
    end
    flag = 0; % to see if there's a 2pi gap between the previous psi and the current one
    y_1 = y_1*sign;

    if abs(psi_P(i)-prev_psi_P)> 2*pi
        flag = 1;
        psi_P = psi_P - 2*pi; % controlla la situa con - o col +, potrebbe esserci un problema se non devo sottrarre ma sommare 2pi
    end
    direction = psi_P(i) - pi/2;
    direction = atan2(sin(direction), cos(direction));
    end

== matlab function simulation_environment_thesis/PHASE 3&4  /PHASE  3/REFERENCE GENERATOR/MATLAB Function6 ==
  data: yaw_ref input; yaw_error output; yaw_est input
function yaw_error = normalize(yaw_ref, yaw_est)

yaw_error = atan2(sin(yaw_ref - yaw_est), cos(yaw_ref - yaw_est));

== matlab function simulation_environment_thesis/PHASE 3&4  /PHASE  3/REFERENCE GENERATOR/MATLAB Function7 ==
  data: v_c input; speed output; beta input; speed_0 input; yaw_dock input
function speed = get_yaw(v_c, beta, speed_0, yaw_dock)

    speed = zeros(4,1);
    speed(3:4) = speed_0(3:4);
    v_tot = norm(speed_0);

    speed(1) = cos(yaw_dock)*(v_tot*cos(beta) - v_c(1)) + sin(yaw_dock)*(v_tot*sin(beta) - v_c(2));
    speed(2) = -sin(yaw_dock)*(v_tot*cos(beta) - v_c(1)) + cos(yaw_dock)*(v_tot*sin(beta) - v_c(2));

    
end

== matlab function simulation_environment_thesis/PHASE 1.22/CONTROLLER1/ANTIWINDUP_HEAVE/MATLAB Function11 ==
  data: e_s_w input; K_t_w input; y output
function y = AWU_heave(e_s_w, K_t_w)
y = K_t_w * e_s_w;

== matlab function simulation_environment_thesis/PHASE 1.24/dynamic + kinematic models1/MATLAB Function9 ==
  data: yaw input; eta_dot output; speed input
function eta_dot = Q(yaw, speed) 
% yaw = orientation in the inertial frame, speed = robot's speed in body frame
% eta_dot = robot's speed in inertial frame

eta_dot = zeros(4,1);
R = [cos(yaw), -sin(yaw), 0;
    sin(yaw), cos(yaw), 0;
    0, 0, 1]; % z_inertial == z_body --> stesso frame, quindi la w corrisponde alla z_dot
eta_dot(1:3) = R*speed(1:3); 
eta_dot(4) = speed(4); % la yaw_dot == r di nuovo perché non ci sono né pitch né roll (stesso discorso della velocità lungo z)

== matlab function simulation_environment_thesis/PHASE 3&4.24/PHASE  4/dynamic + kinematic models1/MATLAB Function ==
  data: angle input; angle output
function angle = normalize(angle)

angle = atan2(sin(angle), cos(angle));

== matlab function simulation_environment_thesis/PHASE 3&4.24/PHASE  4/dynamic + kinematic models1/MATLAB Function7 ==
  data: speed_dot output; speed input; tau input
function speed_dot = dynamic_model(speed, tau) % speed_dot = speed_0_dot + delta_speed_dot = delta_speed_dot

    % speed and speed_dot are in the body frame
    speed_dot = zeros(4,1);
    
    W_plus_B = -0.8339;
    % m = 30;
    % I_z = 4;
    % X_du = -25;
    % Y_dv = -2;
    % Z_dw = - 20;
    % N_dr = -9;
    % X_u = -0.2;
    % Y_v = -55;
    % Z_w = 33; 
    % N_r = -4.14;
    % X_u_u = -20;
    % Y_v_v = -150;
    % Z_w_w = 90; 
    % N_r_r = -6.5;
    m = 30;
    I_z = 5;
    X_du = -25;
    Y_dv = -2;
    Z_dw = - 20;
    N_dr = -10;
    X_u = -0.2;
    Y_v = -55;
    Z_w = 35; 
    N_r = -5;
    X_u_u = -20;
    Y_v_v = -150;
    Z_w_w = 100; 
    N_r_r = -7;
    
    m_u = m - X_du;
    m_v = m - Y_dv;
    m_w = m - Z_dw;
    m_r = I_z - N_dr;
    m_u_v = m_u - m_v;
    
    % SIMPLIFIED MODEL
    
    % surge speed_dot
    speed_dot(1) = (X_u*speed(1) + X_u_u*speed(1)*abs(speed(1) + m_v*speed(2)*speed(4)) + tau(1))/m_u;
    % sway speed_dot
    speed_dot(2) = (Y_v*speed(2) + Y_v_v*speed(2)*abs(speed(2) - m_u*speed(1)*speed(4)) + tau(2))/m_v;
    % heave speed_dot
    speed_dot(3) = (-Z_w*speed(3) - Z_w_w*speed(3)*abs(speed(3)) + tau(3) + W_plus_B)/m_w;
    % yaw speed_dot
    speed_dot(4) = (N_r*speed(4) + N_r_r*speed(4)*abs(speed(4) + m_u_v*speed(1)*speed(2)) + tau(4))/m_r;

== matlab function simulation_environment_thesis/PHASE 3&4  /PHASE  3/p, v_c, psi, r (3D) estimate, from range, v_c, psi, z measurement/MATLAB Function ==
  data: p input; y output
function y = range(p)
    y = sqrt(p(1)^2 + p(2)^2 + p(3)^2);

== matlab function simulation_environment_thesis/PHASE 3&4  /PHASE  3/p, v_c, psi, r (3D) estimate, from range, v_c, psi, z measurement/MATLAB Function10 ==
  data: K_knext output; P_k_k input; P_knext_knext output; R input; A input; Q input; G input; x_k_kprev input
function [K_knext, P_knext_knext] = funct(P_k_k, R, A, Q, G, x_k_kprev)

P_knext_k = A*P_k_k*A' + G*Q*G';
H = zeros(6,8);
H(1,1) = x_k_kprev(1)/sqrt(x_k_kprev(1)^2+x_k_kprev(2)^2+x_k_kprev(3)^2);
H(1,2) = x_k_kprev(2)/sqrt(x_k_kprev(1)^2+x_k_kprev(2)^2+x_k_kprev(3)^2);
H(1,3) = x_k_kprev(3)/sqrt(x_k_kprev(1)^2+x_k_kprev(2)^2+x_k_kprev(3)^2);
H(2,4) = 1;
H(3,5) = 1;
H(4,6) = 1;
H(5,7) = 1;
H(6,3) = 1;
K_knext = P_knext_k*H'/(H*P_knext_k*H'+R);
P_knext_knext = (eye(8)-K_knext*H)*P_knext_k;

== matlab function simulation_environment_thesis/PHASE 3&4  /PHASE  3/p, v_c, psi, r (3D) estimate, from range, v_c, psi, z measurement/MATLAB Function6 ==
  data: x_k_kprev input; y output
function y = pred(x_k_kprev)

y = zeros(6,1);
y(1) =sqrt(x_k_kprev(1)^2 + x_k_kprev(2)^2 + x_k_kprev(3)^2);
y(2:5) = x_k_kprev(4:7);
y(6) = x_k_kprev(3);

== matlab function simulation_environment_thesis/PHASE 3&4  /PHASE  3/p, v_c, psi, r (3D) estimate, from range, v_c, psi, z measurement/MATLAB Function7 ==
  data: A input; u input; x_k_k output
function x_k_k = fcn(A,u)

x_k_k = A*u;

== matlab function simulation_environment_thesis/PHASE 3&4  /PHASE  3/p, v_c, psi, r (3D) estimate, from range, v_c, psi, z measurement/MATLAB Function8 ==
  data: B input; u input; y output
function y = fcn(B,u)

y = B*u;

== matlab function simulation_environment_thesis/PHASE 3&4  /PHASE  3/p, v_c, psi, r (3D) estimate, from range, v_c, psi, z measurement/MATLAB Function9 ==
  data: K input; y_err input; y output
function y = err(K,y_err)

y = K*y_err;

== matlab function simulation_environment_thesis/PHASE 3&4.24/PHASE  4/dynamic + kinematic models1/MATLAB Function9 ==
  data: yaw input; eta_dot output; speed input
function eta_dot = Q(yaw, speed) 
% yaw = orientation in the inertial frame, speed = robot's speed in body frame
% eta_dot = robot's speed in inertial frame

eta_dot = zeros(4,1);
R = [cos(yaw), -sin(yaw), 0;
    sin(yaw), cos(yaw), 0;
    0, 0, 1]; % z_inertial == z_body --> stesso frame, quindi la w corrisponde alla z_dot
eta_dot(1:3) = R*speed(1:3); 
eta_dot(4) = speed(4); % la yaw_dot == r di nuovo perché non ci sono né pitch né roll (stesso discorso della velocità lungo z)

== matlab function simulation_environment_thesis/PHASE 1.22/CONTROLLER1/ANTIWINDUP_HEAVE/MATLAB Function14 ==
  data: in input; y output; K input
function y = fcn(in, K)
y = in*K;

== matlab function simulation_environment_thesis/PHASE 3&4.24/PHASE  4/MATLAB Function7 ==
  data: u input; y output
function y = fcn(u)

y = u;
if abs(u-2*pi)<=0.01
    y = u-2*pi;
elseif abs(u+2*pi)<=0.01
    y = u+2*pi;
end

== matlab function simulation_environment_thesis/PHASE 3&4  /PHASE  4/CONTROLLER4/ANTIWINDUP SURGE/MATLAB Function21 ==
  data: in input; y output; K input
function y = fcn(in, K)
y = in*K;

== matlab function simulation_environment_thesis/PHASE 3&4  /PHASE  4/CONTROLLER4/ANTIWINDUP SURGE/MATLAB Function22 ==
  data: in input; y output; K input
function y = fcn(in, K)
y = in*K;

== matlab function simulation_environment_thesis/PHASE 3&4  /PHASE  4/CONTROLLER4/ANTIWINDUP SURGE/MATLAB Function23 ==
  data: e_s_u input; K_t_u input; y output
function y = AWU_surge(e_s_u, K_t_u)
y = K_t_u * e_s_u;

== matlab function simulation_environment_thesis/PHASE 3&4  /PHASE  4/CONTROLLER4/ANTIWINDUP SWAY/MATLAB Function21 ==
  data: in input; y output; K input
function y = fcn(in, K)
y = in*K;

== matlab function simulation_environment_thesis/PHASE 3&4  /PHASE  4/CONTROLLER4/ANTIWINDUP SWAY/MATLAB Function22 ==
  data: in input; y output; K input
function y = fcn(in, K)
y = in*K;

== matlab function simulation_environment_thesis/PHASE 3&4  /PHASE  4/CONTROLLER4/ANTIWINDUP SWAY/MATLAB Function23 ==
  data: e_s_v input; K_t_v input; y output
function y = AWU_sway(e_s_v, K_t_v)
y = K_t_v * e_s_v;

== matlab function simulation_environment_thesis/PHASE 3&4  /PHASE  4/CONTROLLER4/ANTIWINDUP_HEAVE/MATLAB Function11 ==
  data: e_s_w input; K_t_w input; y output
function y = AWU_heave(e_s_w, K_t_w)
y = K_t_w * e_s_w;

== matlab function simulation_environment_thesis/PHASE 3&4  /PHASE  4/CONTROLLER4/ANTIWINDUP_HEAVE/MATLAB Function14 ==
  data: in input; y output; K input
function y = fcn(in, K)
y = in*K;

== matlab function simulation_environment_thesis/PHASE 3&4  /PHASE  4/CONTROLLER4/ANTIWINDUP_HEAVE/MATLAB Function15 ==
  data: in input; y output; K input
function y = fcn(in, K)
y = in*K;

== matlab function simulation_environment_thesis/MATLAB Function28 ==
  data: stop1 input; state output; stop2 input; out input; out1 input; docked input
function state = STATE_MACHINE(stop1, stop2, out, out1, docked)

    state = 1;
    if stop1 == 1 && stop2 == 0 && docked == 0
            state = 2; % phase 1 is over -> phase 2

    elseif stop1 == 1 && stop2 == 1 && docked == 0
            if out == 0
                state = 3;
                if out1 == 1
                    state = 4;
                end
            else
                state = 4;
            end
    end

== matlab function simulation_environment_thesis/PHASE 1.22/CONTROLLER1/ANTIWINDUP_HEAVE/MATLAB Function15 ==
  data: in input; y output; K input
function y = fcn(in, K)
y = in*K;

== matlab function simulation_environment_thesis/PHASE 3&4  /PHASE  4/CONTROLLER4/ANTIWINDUP_HEAVE/MATLAB Function16 ==
  data: in input; y output; K input
function y = fcn(in, K)
y = in*K;
